simulink model "TosAeb"  (R2021a)
settings: Solver=FixedStepDiscrete  StartTime=0.0  StopTime=inf  FixedStep=0.01  RelTol=1e-3
undefined symbols (not defined in the file or in its folder): ACCModSts, AccSts1, AEB_ObjectMovingOrientation, AEB_ObjectMovingState, CN_BTCActive, CN_CurrentGearR, CN_CurrentGearR_DHT, CN_PTCActive, CN_VDCActive, CN_VSC_EspOff, CN_VSC_FCW_AEB_Error, CN_VSC_FCW_AEB_NoDispay, CN_VSC_FCW_AEB_PerfmDgdtn, CN_VSC_FCW_AEB_TempUnAvlbl, CN_VSC_VehStandstill, CNaccelAvoidMax, CNaccelAvoidMin, CNbrakePedalPress, CNcomfortStrLeftAccelMax, CNcomfortStrLeftAccelMin, CNcomfortStrRightAccelMax, CNcomfortStrRightAccelMin, CNDrvDoorOpen, CNDrvModeEco, CNDrvModeNorm, CNDrvModeOther, CNDrvModeSport, CNDrvSBRreminder, CNNull, CNobjInvalidID, CNPassDoorOpen, CNPassSBRreminder, CNPEBSAccelNegInf, CNPEBSAccelNull, CNPEBSTimeInf, CNPEBSTimeNull, CNSTOPMAX, CNSTOPNULL, CNsubjWidth, CNSwtOFF, CNSwtON, CNTTCMAX, CNTTCNULL, CNVehConfigAEBCycNone, CNVehConfigAEBNone, CNVehConfigAEBPedNone, CNVehConfigFCWNone, CNVehConfigJANone, CollisionSeverity, FALSE, FCWSnvty, JACycObjCalcInfoDefaultParams, JAPedObjCalcInfoDefaultParams, JAVehObjCalcInfoDefaultParams, latCycObjCalcInfoDefaultParams, latPedObjCalcInfoDefaultParams, longObjCalcInfoDefaultParams, ModSts, movingStatus, ObjectType ... (+1112)
libraries: simulink x3031, AEBSFunctionLib x50, Ta_Library x33, AEBSStateMachineLib x23, Mylibrary x12, simulink_extras x7, ObjSelect_lib x3, dspstat3 x2
(part 93 of 102)

== matlab function TosAeb/AEB_Objsel/TA_Processor/AEB_ObjSelect/TargetInfoCheck/Data_precess/TA_EffectCalculate/Obj_FilterFlgCalculate/Lat_ObjFilter/Lat_Objelse/Lat_ObjPre/Subsystem/LatDis_Cal/L2R/L2R_Calculate1 ==
  data: dy input; Valid_Flg output; Width_Large input; Width_half input; dx input; ax input; ay input; vx input; vy input; TTI output; P_TA_PrmForVehLongth input
function [Valid_Flg,TTI] = fcn(dy,Width_Large,Width_half,dx,ax,ay,vx,vy,P_TA_PrmForVehLongth)
%Valid_Flg = false;
%TTI = single(100);
distance_dx = dx;
% 目标处于车辆右侧，无重叠度，向右行驶；
if dy <= single(0) && abs(dy) > Width_Large && vy < single(0)
    Valid_Flg = false;
    TTI = single(100);
% 目标处于车辆右侧，存在重叠，向右行驶
elseif dy <= single(0) && abs(dy) <= Width_Large && vy < single(0)
    distance_y1 = abs(dy) - Width_Large;
    time_1 = solution(single(0.5)*ay,vy,distance_y1);
    distance_x1 = abs(vx)*time_1 + single(0.5)*ax*(time_1)^2;
    time = solution(single(0.5)*ax,vx,distance_dx);
    if distance_x1 <= single(0) || time > time_1
        Valid_Flg = false;
        TTI = single(100);
    else
        if distance_x1 - dx > single(-1) * P_TA_PrmForVehLongth || time <= time_1
            Valid_Flg = true;
            TTI = time;
        else
            Valid_Flg = false;
            TTI = time;
        end
    end
% 目标处于车辆左侧，存在重叠，向右行驶
elseif dy >= single(0) && abs(dy) <= Width_Large && vy < single(0)
    distance_y1 = dy - Width_Large;
    time_1 = solution(single(0.5)*ay,vy,distance_y1);
    distance_x1 = abs(vx)*time_1 + single(0.5)*ax*(time_1)^2;
    time = solution(single(0.5)*ax,vx,distance_dx);
    if distance_x1 <= single(0) || time > time_1
        Valid_Flg = false;
        TTI = single(100);
    else
        if distance_x1 - dx > single(-1)* P_TA_PrmForVehLongth || time <= time_1
            Valid_Flg = true;
            TTI = time;
        else
            Valid_Flg = false;
            TTI = time;
        end
    end
% 目标处于车辆左侧，不存在重叠，向右行驶
elseif dy > single(0) && abs(dy) > Width_Large && vy < single(0)
    distance_y1 = dy - Width_Large;
    distance_y2 = dy + Width_Large;
    time_1 = solution(single(0.5)*ay,vy,distance_y1);
    time_2 = solution(single(0.5)*ay,vy,distance_y2);
    distance_x1 = abs(vx)*time_1 + single(0.5)*ax*(time_1)^2;
    distance_x2 = abs(vx)*time_2 + single(0.5)*ax*(time_2)^2;
    time = solution(single(0.5)*ax,vx,distance_dx);
    if distance_x1 <= single(0) || distance_x2 <= single(0) || time < time_1 -single(1)|| time > time_2 + single(1)
        Valid_Flg = false;
        TTI = single(100);
    else
        if (distance_x1 - dx >= single(0) && distance_x2 - dx <= P_TA_PrmForVehLongth) || (time >= (time_1-single(1)) && time <= (time_2+single(1)))
            Valid_Flg = true;
            TTI = time;
        else
            Valid_Flg = false;
        TTI = single(100);
        end
    end
else
    Valid_Flg = false;
        TTI = single(100);
end
end
%% 一元二次方程求解
function result = solution(a,b,c)
d = single(1) *c;
delta = single(b*b - 4*a*d);
if a == single(0)
    result =  single(c/b);
else
    if delta > single(0)
        r1 = single(((-b) - sqrt(delta))/(2*a));
        r2 = single(((-b) + sqrt(delta))/(2*a));
        if r1 > single(0) && r2 >single(0)
            result = min(r1,r2);
        elseif r1 > single(0) && r2 <= single(0)
            result = r1;
        elseif r1 <= single(0) && r2 > single(0)
            result = r2;
        else
            result = single(100);
        end
    elseif delta == single(0)
        r1 = single((-b)/(2*a));
        r2 = r1;
        if r2 > single(0)
            result = r2;
        else
            result = single(100);
        end
    else
        result = single(100);
    end
end
end
%%

== matlab function TosAeb/AEB_Objsel/TA_Processor/AEB_ObjSelect/TargetInfoCheck/Data_precess/TA_EffectCalculate/Obj_FilterFlgCalculate/Lat_ObjFilter/Lat_Objelse/Lat_ObjPre/Subsystem/LatDis_Cal/R2L/R2L_Calculate ==
  data: dy input; Valid_Flg output; Width_Large input; dx input; ax input; ay input; vx input; vy input; TTI output
function [Valid_Flg,TTI] = fcn(dy,Width_Large,dx,ax,ay,vx,vy)
%Valid_Flg = false;
%TTI = single(100);
distance_dx = dx;
% 目标处于车辆左侧，无重叠度，向左行驶；
if abs(dy) >= Width_Large && dy > single(0) && vy > single(0)
    Valid_Flg = false;
    TTI = single(100);
% 目标处于车辆左侧，存在重叠，向左行驶
elseif dy >= single(0) && abs(dy) < Width_Large && vy > single(0)
    distance_y1 = Width_Large - abs(dy);
    if distance_y1 * vy >single(0)
        distance_y1 = single(-1)*distance_y1;
    else
    end
    time_1 = solution(single(0.5)*ay,vy,distance_y1);
    distance_x1 = abs(vx)*time_1 + single(0.5)*ax*(time_1)^2;
    time = solution(single(0.5)*ax,vx,distance_dx);
    if distance_x1 <= single(0) || time > single(10) || time_1 >single(10)
        Valid_Flg = false;
        TTI = single(100);
    else
       
            Valid_Flg = true;
            TTI = time;
        
    end
% 目标处于车辆右侧，存在重叠，向左行驶
elseif dy <= single(0) && abs(dy) < Width_Large && vy > single(0)
    distance_y1 = Width_Large + dy;
    if distance_y1 * vy >single(0)
        distance_y1 = single(-1)*distance_y1;
    else
    end
    time_1 = solution(single(0.5)*ay,vy,distance_y1);
    distance_x1 = abs(vx)*time_1 + single(0.5)*ax*(time_1)^2;
    time = solution(single(0.5)*ax,vx,distance_dx);
    if distance_x1 <= single(0) || time > single(10) || time_1 >single(10)
        Valid_Flg = false;
        TTI = single(100);
    else
        
            Valid_Flg = true;
            TTI = time;
       
    end
% 目标处于车辆右侧，不存在重叠，向左行驶
elseif dy < single(0) && abs(dy) > Width_Large && vy > single(0)
    distance_y1 = Width_Large + dy;
    distance_y2 = dy - Width_Large;
    time_1 = solution(single(0.5)*ay,vy,distance_y1);
    time_2 = solution(single(0.5)*ay,vy,distance_y2);
    distance_x1 = abs(vx)*time_1 + single(0.5)*ax*(time_1)^2;
    distance_x2 = abs(vx)*time_2 + single(0.5)*ax*(time_2)^2;
    time = solution(single(0.5)*ax,vx,distance_dx);
    if distance_x1 <= single(0) || distance_x2 <= single(0)|| time > single(10) || time_1 >single(10)||time_2 >single(10)
        Valid_Flg = false;
        TTI = single(100);
    else
      
            Valid_Flg = true;
            TTI = time;
        
    end
else
    Valid_Flg = false;
        TTI = single(100);
end
end
%% 一元二次方程求解
function result = solution(a,b,c)
d = single(1) *c;
delta = b*b - 4*a*d;
if abs(a) <= single(0.01) && b ~= single(0)
    result =  abs(c/b);
else
    if delta > single(0)
        r1 = ((-b) - sqrt(delta))/(2*a);
        r2 = ((-b) + sqrt(delta))/(2*a);
        if r1 > single(0) && r2 >single(0)
            result = min(r1,r2);
        elseif r1 > single(0) && r2 <= single(0)
            result = r1;
        elseif r1 <= single(0) && r2 > single(0)
            result = r2;
        else
            result = single(100);
        end
    elseif delta == single(0)
        r1 = (-b)/(2*a);
        r2 = r1;
        if r2 > single(0)
            result = r2;
        else
            result = single(100);
        end
    else
        result = single(100);
    end
end
end
%%

== matlab function TosAeb/AEB_Objsel/TA_Processor/AEB_ObjSelect/TargetInfoCheck/Data_precess/TA_EffectCalculate/Obj_FilterFlgCalculate/Lat_ObjFilter/Lat_Objelse/Lat_ObjPre/Subsystem/LatDis_Cal/R2L/R2L_Calculate1 ==
  data: dy input; Valid_Flg output; Width_Large input; Width_half input; dx input; ax input; ay input; vx input; vy input; TTI output; P_TA_PrmForVehLongth input
function [Valid_Flg,TTI] = fcn(dy,Width_Large,Width_half,dx,ax,ay,vx,vy,P_TA_PrmForVehLongth)
%Valid_Flg = false;
%TTI = single(100);
distance_dx = dx;
% 目标处于车辆左侧，无重叠度，向左行驶；
if abs(dy) >= Width_Large && dy > single(0) && vy > single(0)
    Valid_Flg = false;
    TTI = single(100);
% 目标处于车辆左侧，存在重叠，向左行驶
elseif dy >= single(0) && abs(dy) < Width_Large && vy > single(0)
    distance_y1 = Width_Large - abs(dy);
    time_1 = solution(ay,vy,distance_y1);
    distance_x1 = abs(vx)*time_1 + single(0.5)*ax*(time_1)^2;
    time = solution(ax,vx,distance_dx);
    if distance_x1 <= single(0) || time > time_1
        Valid_Flg = false;
        TTI = single(100);
    else
        if distance_x1 - dx > single(-1) * P_TA_PrmForVehLongth || time <= time_1
            Valid_Flg = true;
            TTI = time;
        else
            Valid_Flg = false;
            TTI = time;
        end
    end
% 目标处于车辆右侧，存在重叠，向左行驶
elseif dy <= single(0) && abs(dy) < Width_Large + single(0.5) && vy > single(0)
    distance_y1 = Width_Large + dy;
    time_1 = solution(ay,vy,distance_y1);
    distance_x1 = abs(vx)*time_1 + single(0.5)*ax*(time_1)^2;
    time = solution(ax,vx,distance_dx);
    if distance_x1 <= single(0) || time > time_1
        Valid_Flg = false;
        TTI = single(100);
    else
        if distance_x1 - dx > single(-1)* P_TA_PrmForVehLongth || time <= time_1
            Valid_Flg = true;
            TTI = time;
        else
            Valid_Flg = false;
            TTI = time;
        end
    end
% 目标处于车辆右侧，不存在重叠，向左行驶
elseif dy < single(0) && abs(dy) > Width_Large && vy > single(0)
    distance_y1 = Width_Large + dy;
    distance_y2 = dy - Width_Large;
    time_1 = solution(ay,vy,distance_y1);
    time_2 = solution(ay,vy,distance_y2);
    distance_x1 = abs(vx)*time_1 + single(0.5)*ax*(time_1)^2;
    distance_x2 = abs(vx)*time_2 + single(0.5)*ax*(time_2)^2;
    time = solution(ax,vx,distance_dx);
    if distance_x1 <= single(0) || distance_x2 <= single(0) || time > time_1 || time < time_2
        Valid_Flg = false;
        TTI = single(100);
    else
       if (distance_x1 - dx >= single(0) && distance_x2 - dx <= P_TA_PrmForVehLongth) || (time <= time_1 && time >= time_2)
            Valid_Flg = true;
            TTI = time;
        else
            Valid_Flg = false;
        TTI = single(100);
        end
    end
else
    Valid_Flg = false;
        TTI = single(100);
end
end
%% 一元二次方程求解
function result = solution(a,b,c)
d = single(1) *c;
delta = b*b - 4*a*d;
if a == single(0)
    result =  c/b;
else
    if delta > single(0)
        r1 = ((-b) - sqrt(delta))/(2*a);
        r2 = ((-b) + sqrt(delta))/(2*a);
        if r1 > single(0) && r2 >single(0)
            result = min(r1,r2);
        elseif r1 > single(0) && r2 <= single(0)
            result = r1;
        elseif r1 <= single(0) && r2 > single(0)
            result = r2;
        else
            result = single(100);
        end
    elseif delta == single(0)
        r1 = (-b)/(2*a);
        r2 = r1;
        if r2 > single(0)
            result = r2;
        else
            result = single(100);
        end
    else
        result = single(100);
    end
end
end
%%

== matlab function TosAeb/AEB_Objsel/TA_Processor/AEB_ObjSelect/TargetInfoCheck/Data_precess/TA_EffectCalculate/Obj_FilterFlgCalculate/Lat_ObjFilter/Lat_Objelse/Lat_ObjPre/Subsystem/LatDis_Cal1/L2R/L2R_Calculate ==
  data: dy input; Valid_Flg output; Width_Large input; Width_half input; dx input; ax input; ay input; vx input; vy input; TTI output; P_TA_PrmForVehLongth input
function [Valid_Flg,TTI] = fcn(dy,Width_Large,Width_half,dx,ax,ay,vx,vy,P_TA_PrmForVehLongth)
%Valid_Flg = false;
%TTI = single(100);
distance_dx = dx;
% 目标处于车辆右侧，无重叠度，向右行驶；
if dy >= Width_Large && vy > single(0)
    Valid_Flg = false;
    TTI = single(100);
% 目标处于车辆右侧，存在重叠，向右行驶
elseif dy >= single(0) && dy < Width_Large && vy > single(0)
    distance_y1 = abs(dy) - Width_Large;
    time_1 = solution(ay,vy,distance_y1);
    distance_x1 = abs(vx)*time_1 + single(0.5)*ax*(time_1)^2;
    time = solution(ax,vx,distance_dx);
    if distance_x1 <= single(0) || time > time_1
        Valid_Flg = false;
        TTI = single(100);
    else
        if distance_x1 - dx > single(-1) * P_TA_PrmForVehLongth || time <= time_1
            Valid_Flg = true;
            TTI = time;
        else
            Valid_Flg = false;
            TTI = time;
        end
    end
% 目标处于车辆左侧，存在重叠，向右行驶
elseif dy <= single(0) && abs(dy) < Width_Large && vy > single(0)
    distance_y1 = dy - Width_Large;
    time_1 = solution(ay,vy,distance_y1);
    distance_x1 = abs(vx)*time_1 + single(0.5)*ax*(time_1)^2;
    time = solution(ax,vx,distance_dx);
    if distance_x1 <= single(0) || time > time_1
        Valid_Flg = false;
        TTI = single(100);
    else
        if distance_x1 - dx > single(-1)* P_TA_PrmForVehLongth || time <= time_1
            Valid_Flg = true;
            TTI = time;
        else
            Valid_Flg = false;
            TTI = time;
        end
    end
% 目标处于车辆左侧，不存在重叠，向右行驶
elseif dy < single(0) && abs(dy) > Width_Large && vy > single(0)
    distance_y1 = dy - Width_Large;
    distance_y2 = dy + Width_Large;
    time_1 = solution(ay,vy,distance_y1);
    time_2 = solution(ay,vy,distance_y2);
    distance_x1 = abs(vx)*time_1 + single(0.5)*ax*(time_1)^2;
    distance_x2 = abs(vx)*time_2 + single(0.5)*ax*(time_2)^2;
    time = solution(ax,vx,distance_dx);
    if distance_x1 <= single(0) || distance_x2 <= single(0) || time > time_1 || time < time_2
        Valid_Flg = false;
        TTI = single(100);
    else
        if (distance_x1 - dx >= single(0) && distance_x2 - dx <= P_TA_PrmForVehLongth) || (time <= time_1 && time >= time_2)
            Valid_Flg = true;
            TTI = time;
        else
            Valid_Flg = false;
        TTI = single(100);
        end
    end
else
    Valid_Flg = false;
        TTI = single(100);
end
end
%% 一元二次方程求解
function result = solution(a,b,c)
d = single(1) *c;
delta = single(b*b - 4*a*d);
if a == single(0)
    result =  single(c/b);
else
    if delta > single(0)
        r1 = single(((-b) - sqrt(delta))/(2*a));
        r2 = single(((-b) + sqrt(delta))/(2*a));
        if r1 > single(0) && r2 >single(0)
            result = min(r1,r2);
        elseif r1 > single(0) && r2 <= single(0)
            result = r1;
        elseif r1 <= single(0) && r2 > single(0)
            result = r2;
        else
            result = single(100);
        end
    elseif delta == single(0)
        r1 = single((-b)/(2*a));
        r2 = r1;
        if r2 > single(0)
            result = r2;
        else
            result = single(100);
        end
    else
        result = single(100);
    end
end
end
%%

== stateflow chart TosAeb/FcwAebJa_Step_sys/ALG_AEBSystem/ALG_AEBController/AEB_FuncImpManagementApp/FuncManeuArbCalc/brkFuncManeuArbCalc/axTgtReqValueCalc1/axTgtReqValueCalc4AebControl/JerkFuncIncCalc/IncPhaseCalc/Chart ==
  state IncrementStage [function]
    (default) -> (junction 173)
    (junction 173) -> (junction 167) [order 1] : [aErrorDesiredTgtValue < 0]
    (junction 167) -> (junction 175)
    (junction 173) -> (junction 179) [order 2] : {
        |    time4IncControlling = 0;
        |   currentIncStage = 0;
        | }
    (junction 175) -> (junction 171) [order 1] : [aErrorDesiredTgtValue <...
        | P_AEB_FIM_aErrorThres4BrakeStage5]
    (junction 175) -> (junction 170) [order 2]
    (junction 171) -> (junction 166) : {
        |   setStage(5);
        |   currentIncStage = 5;
        | }
    (junction 170) -> (junction 174) [order 1] : [aErrorDesiredTgtValue <...
        |  P_AEB_FIM_aErrorThres4BrakeStage4]
    (junction 170) -> (junction 168) [order 2]
    (junction 174) -> (junction 172) : {
        |    setStage(4);
        |    currentIncStage = 4;
        | }
    (junction 168) -> (junction 150) [order 1] : [aErrorDesiredTgtValue <...
        |  P_AEB_FIM_aErrorThres4BrakeStage3]
    (junction 168) -> (junction 169) [order 2]
    (junction 150) -> (junction 151) : {
        |    setStage(3);
        |    currentIncStage = 3;
        | }
    (junction 169) -> (junction 138) [order 1] : [aErrorDesiredTgtValue <...
        |  P_AEB_FIM_aErrorThres4BrakeStage2]
    (junction 169) -> (junction 146) [order 2]
    (junction 138) -> (junction 137) : {
        |    setStage(2);
        |    currentIncStage = 2;
        | }
    (junction 146) -> (junction 141) [order 1] : [aErrorDesiredTgtValue <...
        |  P_AEB_FIM_aErrorThres4BrakeStage1]
    (junction 146) -> (junction 145) [order 2]
    (junction 141) -> (junction 143) : {
        |    setStage(1) ;
        |    currentIncStage = 1;
        | }
    (junction 145) -> (junction 179)
    (junction 143) -> (junction 145)
    (junction 137) -> (junction 143)
    (junction 151) -> (junction 137)
    (junction 172) -> (junction 151)
    (junction 166) -> (junction 172)
    (junction 179) -> (junction 178)
  state setStage(Stage) [function]
    (default) -> (junction 183)
    (junction 183) -> (junction 185) [order 1] : [Stage > ReachedIncStage]
    (junction 185) -> (junction 187) : {
        |   ReachedIncStage = Stage;
        | }
    (junction 183) -> (junction 189) [order 2]
    (junction 187) -> (junction 189)
    (junction 189) -> (junction 192)
  state IncrementTimer(CountStage) [function]
    (default) -> (junction 92)
    (junction 92) -> (junction 98) : {
        |   index=0;
        | }
    (junction 98) -> (junction 94) [order 1] : [index<CountStage]
    (junction 97) -> (junction 98)
    (junction 98) -> (junction 96) [order 2]
    (junction 94) -> (junction 95) : {
        |    time4IncControlling += Ts;
        | }
    (junction 95) -> (junction 97) : {index=index+1;}
  (default) -> (junction 199)
  (junction 199) -> (junction 202) [order 1] : [AebActiveFirstCycle == true]
  (junction 199) -> (junction 201) [order 2]
  (junction 202) -> (junction 205) : {
      |    ReachedIncStage = 0;
      |    currentIncStage = 0;
      |    time4IncControlling = 0;
      | }
  (junction 205) -> (junction 201)
  (junction 201) -> (junction 53) : {
      |    IncrementStage();
      | }
  (junction 53) -> (junction 58) [order 1] : [ReachedIncStage > ...
      | currentIncStage]
  (junction 53) -> (junction 62) [order 2] : {
      |   IncrementTimer(currentIncStage);
      | }
  (junction 58) -> (junction 60)
  (junction 60) -> (junction 62)
  (junction 62) -> (junction 65)
  (junction 65) -> (junction 101) [order 1] : [dxFrontBumperPredicted < P_AEB_FIM_distMinThres4LowControlling]
  (junction 65) -> (junction 105) [order 2]
  (junction 101) -> (junction 103)
  (junction 103) -> (junction 110) [order 1] : [aErrorDesiredTgtValue < P_AEB_FIM_aDeltaThres4BrakeStage6]
  (junction 103) -> (junction 115) [order 2]
  (junction 110) -> (junction 113) : {
      |   IncrementTimer(uint8(2*P_AEB_FIM_TimerNumber4JerkInc));
      | }
  (junction 115) -> (junction 117) [order 1] : [aErrorDesiredTgtValue < P_AEB_FIM_aErrorThres4BrakeStage5]
  (junction 115) -> (junction 122) [order 2]
  (junction 117) -> (junction 119) : {
      |    IncrementTimer(P_AEB_FIM_TimerNumber4JerkInc);
      | }
  (junction 122) -> (junction 105)
  (junction 119) -> (junction 122)
  (junction 113) -> (junction 119)
  (junction 105) -> (junction 108)
  data: Stage function_input uint8; CountStage function_input uint8; aErrorDesiredTgtValue input; dxFrontBumperPredicted input; AebActiveFirstCycle input; Ts input; P_AEB_FIM_aErrorThres4BrakeStage1 input; P_AEB_FIM_aErrorThres4BrakeStage2 input; P_AEB_FIM_aErrorThres4BrakeStage3 input; P_AEB_FIM_aErrorThres4BrakeStage4 input; P_AEB_FIM_aErrorThres4BrakeStage5 input; ReachedIncStage output uint8 =0; currentIncStage output uint8 =0; time4IncControlling output single =0; index local uint8; P_AEB_FIM_aDeltaThres4BrakeStage6 input; P_AEB_FIM_distMinThres4LowControlling input; P_AEB_FIM_TimerNumber4JerkInc input

== stateflow chart TosAeb/FcwAebJa_Step_sys/ALG_AEBSystem/ALG_AEBController/AEB_FuncImpManagementApp/FuncManeuArbCalc/brkFuncManeuArbCalc/axTgtReqValueCalc1/axTgtReqValueCalc4AebControl/aDesiredCalc/If Action Subsystem1/accelAvoidLowLvlBrkCalc/AccelAvoidSituationJudge ==
  (default) -> (junction 16)
  (junction 16) -> (junction 17) [order 1] : [vx < 0]
  (junction 16) -> (junction 27) [order 2]
  (junction 17) -> (junction 74)
  (junction 74) -> (junction 76) [order 1] : [axAbsObj  < 0]
  (junction 27) -> (junction 29) [order 1] : [ axAbsObj  < 0]
  (junction 74) -> (junction 25) [order 2] : {
      | 	send(Situation2);
      | }
  (junction 76) -> (junction 19) : {
      | 	send(Situation1);
      | }
  (junction 27) -> (junction 33) [order 2] : {
      | 	send(Situation4);
      | }
  (junction 29) -> (junction 31) : {
      | 	send(Situation3);
      | }
  (junction 31) -> (junction 33)
  (junction 25) -> (junction 31)
  (junction 19) -> (junction 25)
  (junction 33) -> (junction 86)
  data: vx input; axAbsObj input
  events: Situation1 output FUNCTION_CALL_EVENT; Situation2 output FUNCTION_CALL_EVENT; Situation3 output FUNCTION_CALL_EVENT; Situation4 output FUNCTION_CALL_EVENT

== stateflow chart TosAeb/FcwAebJa_Step_sys/ALG_AEBSystem/ALG_AEBController/AEB_FuncImpManagementApp/FuncManeuArbCalc/brkFuncManeuArbCalc/axTgtReqValueCalc1/axTgtReqValueCalc4AebControl/aDesiredCalc/If Action Subsystem1/tObjStopCalc/StopTimeFlow ==
  state temptStop = tStopFuncCalc(velRel,accelRel) [function]
  (default) -> (junction 2)
  (junction 2) -> (junction 3) [order 1] : [accelRel == 0]
  (junction 2) -> (junction 14) [order 2]
  (junction 3) -> (junction 5)
  (junction 14) -> (junction 16) [order 1] : [(accelRel > 0 && velRel <= 0)||...
      | (accelRel < 0 && velRel >= 0)]
  (junction 5) -> (junction 7) [order 1] : [velRel == 0]
  (junction 14) -> (junction 20) [order 2] : {
      | 	tStop = CNSTOPMAX;
      |  }
  (junction 16) -> (junction 18) : {
      |
      | 	tStop = tStopFuncCalc(velRel,accelRel);
      | }
  (junction 5) -> (junction 11) [order 2] : {
      | 	tStop = CNSTOPMAX;
      | }
  (junction 7) -> (junction 9) : {
      | 	tStop = CNNull;
      | }
  (junction 18) -> (junction 20)
  (junction 11) -> (junction 18)
  (junction 9) -> (junction 11)
  (junction 20) -> (junction 24)
  data: velRel function_input; accelRel function_input; temptStop function_output; tStop output; CNNull parameter double; CNSTOPMAX parameter double; accelRel input; velRel input

== stateflow chart TosAeb/FcwAebJa_Step_sys/ALG_AEBSystem/ALG_AEBController/AEB_FuncImpManagementApp/FuncManeuArbCalc/brkFuncManeuArbCalc/axTgtReqValueCalc1/axTgtReqValueCalc4AebControl/axTgtReqValueCalc/axTgtReqValueFinal/axTgtReqValueCalc/axTgtReqValueCalc/axTgtReqValueFlow ==
  (default) -> (junction 7)
  (junction 7) -> (junction 9) [order 1] : [abs(aErrorADesiredAxTgtReq) <= 1e-6]
  (junction 9) -> (junction 11) : {
      |    axTgtReqValue = ...
      |        accelAvoid;
      | }
  (junction 7) -> (junction 21) [order 2]
  (junction 21) -> (junction 23) [order 1] : [aErrorADesiredAxTgtReq < 0]
  (junction 21) -> (junction 25) [order 2]
  (junction 23) -> (junction 13)
  (junction 13) -> (junction 15) [order 1] : [aErrorADesiredAxTgtReq < JerkDecTimeSlot]
  (junction 13) -> (junction 19) [order 2] : {
      |   axTgtReqValue = ...
      |         axTgtReqValueK1 + aErrorADesiredAxTgtReq;
      | }
  (junction 15) -> (junction 17) : {
      |    axTgtReqValue = ...
      |          axTgtReqValueK1 + JerkDecTimeSlot;
      | }
  (junction 25) -> (junction 27) [order 1] : [aErrorADesiredAxTgtReq > JerkIncTimeSlot]
  (junction 25) -> (junction 31) [order 2] : {
      |   axTgtReqValue =...
      |         axTgtReqValueK1 + aErrorADesiredAxTgtReq;
      | }
  (junction 27) -> (junction 29) : {
      |   axTgtReqValue = ...
      |         axTgtReqValueK1 + JerkIncTimeSlot;
      | }
  (junction 11) -> (junction 17)
  (junction 29) -> (junction 31)
  (junction 19) -> (junction 29)
  (junction 17) -> (junction 19)
  (junction 31) -> (junction 38)
  (junction 38) -> (junction 40) [order 1] : [accelMax < accelMin]
  (junction 38) -> (junction 44) [order 2] : {
      | axTgtReqValue = min(max(axTgtReqValue, accelMin), accelMax);
      | }
  (junction 40) -> (junction 42) : {
      |   axTgtReqValue = accelMin;
      | }
  (junction 42) -> (junction 44)
  (junction 44) -> (junction 49)
  data: JerkIncTimeSlot input; JerkDecTimeSlot input; aErrorADesiredAxTgtReq input; accelAvoid input; accelMax input; accelMin input; axTgtReqValueK1 input; axTgtReqValue output

== stateflow chart TosAeb/FcwAebJa_Step_sys/ALG_AEBSystem/ALG_AEBController/AEB_FuncImpManagementApp/FuncManeuArbCalc/brkFuncManeuArbCalc/axTgtReqValueCalc1/axTgtReqValueCalc4AebControl/axTgtReqValueCalc/axTgtReqValueFinal1/axTgtReqValueCalc/axTgtReqValueCalc/axTgtReqValueFlow ==
  (default) -> (junction 7)
  (junction 7) -> (junction 9) [order 1] : [abs(aErrorADesiredAxTgtReq) <= 1e-6]
  (junction 9) -> (junction 11) : {
      |    axTgtReqValue = ...
      |        accelAvoid;
      | }
  (junction 7) -> (junction 21) [order 2]
  (junction 21) -> (junction 23) [order 1] : [aErrorADesiredAxTgtReq < 0]
  (junction 21) -> (junction 25) [order 2]
  (junction 23) -> (junction 13)
  (junction 13) -> (junction 15) [order 1] : [aErrorADesiredAxTgtReq < JerkDecTimeSlot]
  (junction 13) -> (junction 19) [order 2] : {
      |   axTgtReqValue = ...
      |         axTgtReqValueK1 + aErrorADesiredAxTgtReq;
      | }
  (junction 15) -> (junction 17) : {
      |    axTgtReqValue = ...
      |          axTgtReqValueK1 + JerkDecTimeSlot;
      | }
  (junction 25) -> (junction 27) [order 1] : [aErrorADesiredAxTgtReq > JerkIncTimeSlot]
  (junction 25) -> (junction 31) [order 2] : {
      |   axTgtReqValue =...
      |         axTgtReqValueK1 + aErrorADesiredAxTgtReq;
      | }
  (junction 27) -> (junction 29) : {
      |   axTgtReqValue = ...
      |         axTgtReqValueK1 + JerkIncTimeSlot;
      | }
  (junction 11) -> (junction 17)
  (junction 29) -> (junction 31)
  (junction 19) -> (junction 29)
  (junction 17) -> (junction 19)
  (junction 31) -> (junction 38)
  (junction 38) -> (junction 40) [order 1] : [accelMax < accelMin]
  (junction 38) -> (junction 44) [order 2] : {
      | axTgtReqValue = min(max(axTgtReqValue, accelMin), accelMax);
      | }
  (junction 40) -> (junction 42) : {
      |   axTgtReqValue = accelMin;
      | }
  (junction 42) -> (junction 44)
  (junction 44) -> (junction 49)
  data: JerkIncTimeSlot input; JerkDecTimeSlot input; aErrorADesiredAxTgtReq input; accelAvoid input; accelMax input; accelMin input; axTgtReqValueK1 input; axTgtReqValue output

== stateflow chart TosAeb/FcwAebJa_Step_sys/ALG_AEBSystem/ALG_AEBController/AEB_FuncImpManagementApp/FuncManeuArbCalc/brkFuncManeuArbCalc/axTgtReqValueCalc1/axTgtReqValueCalc4AebFull/BrkFuncDataManagement ==
  state axTgtReqValue = AEB_TgtDecelFunc(maxDecel,maxDecelGredient,axTgtReqValueK1) [function]
  state MaxDecel = VehAEBfullMaxDecelLookupTable(subjVel) [function]
  state MaxDecel = VehAEBpartMaxDecelLookupTable(subjVel) [function]
  state LongFuncAEBTrigger [function]
    (default) -> (junction 231)
    (junction 231) -> (junction 234) [order 1] : [AEBpartFuncImpArb == ModSts.ActiveState]
    (junction 231) -> (junction 232) [order 2]
    (junction 234) -> (junction 242) : {
        | 	maxDecel = P_FIM_VehAEBPartDecelMinThres;
        | 	maxDecelGredient = P_FIM_VehAEBPartDecelGradientThres;
        | 	axTgtReqValue = AEB_TgtDecelFunc(maxDecel,maxDecelGredient,axTgtReqValueK1);
        | }
    (junction 242) -> (junction 236)
    (junction 236) -> (junction 237)
    (junction 232) -> (junction 241) [order 1] : [(AEBfullFuncImpArb == ModSts.ActiveState&&...
        | P_AEB_FIM_BrkControllerConfig == false)]
    (junction 232) -> (junction 254) [order 2]
    (junction 241) -> (junction 239)
    (junction 239) -> (junction 240) [order 1] : [longTgt_MovingState == AEB_ObjectMovingState.Still]
    (junction 240) -> (junction 233) : {
        | 	maxDecel = P_FIM_VehAEBfullDecelMinThresSO;
        | 	maxDecelGredient = ...
        |       P_FIM_VehAEBfullDecelGradientThresSO;
        | }
    (junction 239) -> (junction 235) [order 2] : {
        |
        | 	maxDecel =  ...
        | 	VehAEBfullMaxDecelLookupTable(subjVel);
        | 	maxDecelGredient = ...
        |       P_FIM_VehAEBfullDecelGradientThresMO;
        | }
    (junction 233) -> (junction 235)
    (junction 235) -> (junction 243) : {
        | 	axTgtReqValue = AEB_TgtDecelFunc(maxDecel,maxDecelGredient,axTgtReqValueK1);
        | }
    (junction 243) -> (junction 237)
    (junction 237) -> (junction 250)
    (junction 254) -> (junction 244) [order 1] : [EBAFuncImpArb == ModSts.ActiveState]
    (junction 254) -> (junction 273) [order 2]
    (junction 244) -> (junction 257) : {
        | 	maxDecel = P_FIM_VehEBADecelMinThres;
        | 	maxDecelGredient = P_FIM_VehEBADecelGradientThres;
        | 	axTgtReqValue = AEB_TgtDecelFunc(maxDecel,maxDecelGredient,axTgtReqValueK1);
        | }
    (junction 257) -> (junction 250)
    (junction 250) -> (junction 274)
    (junction 274) -> (junction 273)
    (junction 273) -> (junction 276)
  state LatFuncTrigger [function]
    (default) -> (junction 287)
    (junction 287) -> (junction 281) [order 1] : [cycAEBpartFuncImpArb == ModSts.ActiveState]
    (junction 287) -> (junction 284) [order 2]
    (junction 281) -> (junction 282) : {
        | 	maxDecel = VehAEBpartMaxDecelLookupTable(subjVel);
        | 	maxDecelGredient = P_FIM_CycAEBPartDecelGradientThres;
        | 	axTgtReqValue = AEB_TgtDecelFunc(maxDecel,maxDecelGredient,axTgtReqValueK1);
        | }
    (junction 282) -> (junction 290)
    (junction 290) -> (junction 292)
    (junction 284) -> (junction 285) [order 1] : [cycAEBfullFuncImpArb == ModSts.ActiveState]
    (junction 284) -> (junction 288) [order 2]
    (junction 285) -> (junction 286) : {
        | 	maxDecel = P_FIM_CycAEBfullDecelMinThres;
        | 	maxDecelGredient = P_FIM_CycAEBfullDecelGradientThres;
        | 	axTgtReqValue = AEB_TgtDecelFunc(maxDecel,maxDecelGredient,axTgtReqValueK1);
        | }
    (junction 286) -> (junction 292)
    (junction 292) -> (junction 298)
    (junction 288) -> (junction 289) [order 1] : [pedAEBfullFuncImpArb == ModSts.ActiveState]
    (junction 288) -> (junction 320) [order 2]
    (junction 289) -> (junction 291) : {
        | 	maxDecel = P_FIM_PedAEBfullDecelMinThres;
        | 	maxDecelGredient = P_FIM_PedAEBfullDecelGradientThres;
        | 	axTgtReqValue = AEB_TgtDecelFunc(maxDecel,maxDecelGredient,axTgtReqValueK1);
        | }
    (junction 291) -> (junction 441)
    (junction 441) -> (junction 320)
    (junction 298) -> (junction 441)
    (junction 320) -> (junction 324)
  state FCTBFuncTrigger [function]
    (default) -> (junction 425)
    (junction 425) -> (junction 431) [order 1] : [FCTBFuncImpArb == ModSts.ActiveState]
    (junction 425) -> (junction 432) [order 2]
    (junction 431) -> (junction 443) : {
        | 	%maxDecel = P_FIM_FCTBDecelMinThres;
        | 	%maxDecelGredient = P_FIM_FCTBDecelGradientThres;
        | 	axTgtReqValue = FCTB_BrkgReqVal;
        | }
    (junction 443) -> (junction 424)
    (junction 424) -> (junction 432)
    (junction 432) -> (junction 438)
  state JaFuncTrigger [function]
    (default) -> (junction 365)
    (junction 365) -> (junction 362) [order 1] : [JA_PTW_AEBFuncImpArb == ModSts.ActiveState]
    (junction 365) -> (junction 367) [order 2]
    (junction 362) -> (junction 361)
    (junction 361) -> (junction 385) : {
        | 	maxDecel = P_FIM_JA_PTW_AEBDecelMinThres;
        | 	maxDecelGredient = P_FIM_JA_PTW_AEBDecelGradientThres;
        | 	axTgtReqValue = AEB_TgtDecelFunc(maxDecel,maxDecelGredient,axTgtReqValueK1);
        | }
    (junction 385) -> (junction 366)
    (junction 367) -> (junction 363) [order 1] : [JA_cycAEBFuncImpArb == ModSts.ActiveState]
    (junction 367) -> (junction 359) [order 2]
    (junction 363) -> (junction 358)
    (junction 358) -> (junction 366) : {
        | 	maxDecel = P_FIM_JA_cycAEBDecelMinThres;
        | 	maxDecelGredient = P_FIM_JA_cycAEBDecelGradientThres;
        | 	axTgtReqValue = AEB_TgtDecelFunc(maxDecel,maxDecelGredient,axTgtReqValueK1);
        | }
    (junction 366) -> (junction 360)
    (junction 359) -> (junction 368) [order 1] : [JA_pedAEBFuncImpArb == ModSts.ActiveState]
    (junction 359) -> (junction 453) [order 2]
    (junction 368) -> (junction 364)
    (junction 364) -> (junction 360) : {
        | 	maxDecel = P_FIM_JA_pedAEBDecelMinThres;
        | 	maxDecelGredient = P_FIM_JA_pedAEBDecelGradientThres;
        | 	axTgtReqValue = AEB_TgtDecelFunc(maxDecel,maxDecelGredient,axTgtReqValueK1);
        | }
    (junction 360) -> (junction 447)
    (junction 453) -> (junction 448) [order 1] : [JA_AEBfullFuncImpArb == ModSts.ActiveState]
    (junction 453) -> (junction 386) [order 2]
    (junction 448) -> (junction 449) : {
        | 	maxDecel = P_FIM_JAAEBfullDecelMinThres;
        | 	maxDecelGredient = P_FIM_JAAEBfullDecelGradientThres;
        | 	axTgtReqValue = AEB_TgtDecelFunc(maxDecel,maxDecelGredient,axTgtReqValueK1);
        | }
    (junction 449) -> (junction 447)
    (junction 447) -> (junction 387)
    (junction 387) -> (junction 386)
    (junction 386) -> (junction 390)
  (default) -> (junction 44)
  (junction 44) -> (junction 45) [order 1] : [AEBpartFuncImpArb == ModSts.ActiveState||...
      | (AEBfullFuncImpArb == ModSts.ActiveState&&...
      | P_AEB_FIM_BrkControllerConfig == false)||...
      | EBAFuncImpArb == ModSts.ActiveState]
  (junction 44) -> (junction 48) [order 2]
  (junction 45) -> (junction 46) : {
      | 	LongFuncAEBTrigger();
      | }
  (junction 46) -> (junction 47)
  (junction 47) -> (junction 51)
  (junction 48) -> (junction 49) [order 1] : [cycAEBpartFuncImpArb == ModSts.ActiveState||...
      | cycAEBfullFuncImpArb == ModSts.ActiveState||...
      | pedAEBfullFuncImpArb == ModSts.ActiveState]
  (junction 48) -> (junction 56) [order 2]
  (junction 49) -> (junction 50) : {
      | 	LatFuncTrigger();
      | }
  (junction 50) -> (junction 51)
  (junction 51) -> (junction 59)
  (junction 56) -> (junction 57) [order 1] : [FCTBFuncImpArb == ModSts.ActiveState &&...
      | IsNeedBrake == true]
  (junction 56) -> (junction 192) [order 2]
  (junction 57) -> (junction 58) : {
      | 	FCTBFuncTrigger();
      | }
  (junction 192) -> (junction 194) [order 1] : [JA_PTW_AEBFuncImpArb == ModSts.ActiveState||...
      | JA_cycAEBFuncImpArb == ModSts.ActiveState||...
      | JA_pedAEBFuncImpArb == ModSts.ActiveState||...
      | JA_AEBfullFuncImpArb == ModSts.ActiveState]
  (junction 192) -> (junction 60) [order 2] : {
      | 	maxDecel = 0;
      | 	maxDecelGredient = 0;
      | 	axTgtReqValue = 0;
      |
      | }
  (junction 194) -> (junction 196)
  (junction 58) -> (junction 59)
  (junction 59) -> (junction 198)
  (junction 196) -> (junction 198) : {
      | 	JaFuncTrigger();
      | }
  (junction 198) -> (junction 88)
  (junction 88) -> (junction 60)
  (junction 60) -> (junction 62)
  data: maxDecel function_input single; maxDecelGredient function_input single; axTgtReqValue function_output; axTgtReqValueK1 function_input single; subjVel function_input single; MaxDecel function_output; subjVel function_input single; MaxDecel function_output; maxDecelGredient output single; AEBpartFuncImpArb input Enum: ModSts; cycAEBpartFuncImpArb input Enum: ModSts; AEBfullFuncImpArb input Enum: ModSts; JA_AEBfullFuncImpArb input Enum: ModSts; cycAEBfullFuncImpArb input Enum: ModSts; pedAEBfullFuncImpArb input Enum: ModSts; EBAFuncImpArb input Enum: ModSts; FCTBFuncImpArb input Enum: ModSts; JA_PTW_AEBFuncImpArb input Enum: ModSts; JA_cycAEBFuncImpArb input Enum: ModSts; JA_pedAEBFuncImpArb input Enum: ModSts; subjVel input single; longTgt_MovingState input Enum: AEB_ObjectMovingState; IsNeedBrake input; FCTB_BrkgReqVal input; axTgtReqValueK1 input single; maxDecel output single; axTgtReqValue output single; P_FIM_VehAEBPartDecelGradientThres input; P_FIM_VehAEBPartDecelMinThres input; P_FIM_CycAEBPartDecelGradientThres input; P_FIM_VehAEBfullDecelGradientThresMO input; P_FIM_VehAEBfullDecelGradientThresSO input; P_FIM_VehAEBfullDecelMinThresSO input; P_FIM_CycAEBfullDecelGradientThres input; P_FIM_CycAEBfullDecelMinThres input; P_FIM_PedAEBfullDecelGradientThres input; P_FIM_PedAEBfullDecelMinThres input; P_FIM_FCTBDecelGradientThres input; P_FIM_FCTBDecelMinThres input; P_FIM_JAAEBfullDecelGradientThres input; P_FIM_JAAEBfullDecelMinThres input; P_FIM_VehEBADecelGradientThres input; P_FIM_VehEBADecelMinThres input; P_FIM_JA_PTW_AEBDecelGradientThres input; P_FIM_JA_PTW_AEBDecelMinThres input; P_FIM_JA_cycAEBDecelGradientThres input; P_FIM_JA_cycAEBDecelMinThres input; P_FIM_JA_pedAEBDecelGradientThres input; P_FIM_JA_pedAEBDecelMinThres input; P_AEB_FIM_BrkControllerConfig input

== stateflow chart TosAeb/FcwAebJa_Step_sys/ALG_AEBSystem/ALG_AEBController/AEB_FuncImpManagementApp/FuncManeuArbCalc/brkFuncManeuArbCalc/axTgtReqValueManeuCalc/AEBTriggerManeuCalc1 ==
  state AEBQuitState
    en:
    timeCounter = 0;
    AEBTriggerManeu = true;
    %axTgtReqValueIntial = axTgtReqValue;
    du:
    AEBTriggerManeu = true;
    timeCounter += Ts;
    axTgtReqValueManeu = axTgtReqValueOptCalc(Ts,axJerkQuit,axTgtReqValueIntial);
  state AEBNormalState
    en,du:
    timeCounter = 0;
    AEBTriggerManeu= AEBTrigger;
    axTgtReqValueManeu = axTgtReqValue;
    exit:
    axTgtReqValueIntial = axTgtReqValueK1;
  state axTgtReqValueManeu = axTgtReqValueOptCalc(Ts,axJerkQuit,axTgtReqValueIntial) [function]
  (default) -> (junction 7)
  (junction 7) -> AEBNormalState [order 2]
  (junction 7) -> AEBQuitState [order 1] : [AEBQuitFlg == true]
  AEBQuitState -> AEBNormalState : [timeCounter >= PmaxQuitTime || ...
      | abs(axTgtReqValueManeu) < 1e-6]
  AEBNormalState -> AEBQuitState : [AEBQuitFlg == true]
  data: Ts function_input single; axJerkQuit function_input single; axTgtReqValueIntial function_input single; axTgtReqValueManeu function_output; AEBQuitFlg input; AEBTrigger input; axTgtReqValue input single; axTgtReqValueK1 input single; Ts input single; timeCounter local single; PmaxQuitTime input single; AEBTriggerManeu output boolean; axTgtReqValueManeu output single; axTgtReqValueIntial local single; axJerkQuit input single

== matlab function TosAeb/AEB_Objsel/TA_Processor/AEB_ObjSelect/TargetInfoCheck/Data_precess/TA_EffectCalculate/Obj_FilterFlgCalculate/Lat_ObjFilter/Lat_Objelse/Lat_ObjPre/Subsystem/LatDis_Cal1/R2L/R2L_Calculate ==
  data: dy input; Valid_Flg output; Width_Large input; Width_half input; dx input; ax input; ay input; vx input; vy input; TTI output; P_TA_PrmForVehLongth input
function [Valid_Flg,TTI] = fcn(dy,Width_Large,Width_half,dx,ax,ay,vx,vy,P_TA_PrmForVehLongth)
%Valid_Flg = false;
%TTI = single(100);
distance_dx = dx;
% 目标处于车辆左侧，无重叠度，向左行驶；
if abs(dy) >= Width_Large && dy < single(0) && vy > single(0)
    Valid_Flg = false;
    TTI = single(100);
% 目标处于车辆左侧，存在重叠，向左行驶
elseif dy <= single(0) && abs(dy) < Width_Large && vy > single(0)
    distance_y1 = Width_Large - abs(dy);
    time_1 = solution(ay,vy,distance_y1);
    distance_x1 = abs(vx)*time_1 + single(0.5)*ax*(time_1)^2;
    time = solution(ax,vx,distance_dx);
    if distance_x1 <= single(0) || time > time_1
        Valid_Flg = false;
        TTI = single(100);
    else
        if distance_x1 - dx > single(-1) * P_TA_PrmForVehLongth || time <= time_1
            Valid_Flg = true;
            TTI = time;
        else
            Valid_Flg = false;
            TTI = time;
        end
    end
% 目标处于车辆右侧，存在重叠，向左行驶
elseif dy >= single(0) && dy < Width_Large && vy > single(0)
    distance_y1 = Width_Large + dy;
    time_1 = solution(ay,vy,distance_y1);
    distance_x1 = abs(vx)*time_1 + single(0.5)*ax*(time_1)^2;
    time = solution(ax,vx,distance_dx);
    if distance_x1 <= single(0) || time > time_1
        Valid_Flg = false;
        TTI = single(100);
    else
        if distance_x1 - dx > single(-1)* P_TA_PrmForVehLongth || time <= time_1
            Valid_Flg = true;
            TTI = time;
        else
            Valid_Flg = false;
            TTI = time;
        end
    end
% 目标处于车辆右侧，不存在重叠，向左行驶
elseif dy > single(0) && abs(dy) > Width_Large && vy > single(0)
    distance_y1 = Width_Large + dy;
    distance_y2 = dy - Width_Large;
    time_1 = solution(ay,vy,distance_y1);
    time_2 = solution(ay,vy,distance_y2);
    distance_x1 = abs(vx)*time_1 + single(0.5)*ax*(time_1)^2;
    distance_x2 = abs(vx)*time_2 + single(0.5)*ax*(time_2)^2;
    time = solution(ax,vx,distance_dx);
    if distance_x1 <= single(0) || distance_x2 <= single(0) || time > time_1 || time < time_2
        Valid_Flg = false;
        TTI = single(100);
    else
       if (distance_x1 - dx >= single(0) && distance_x2 - dx <= P_TA_PrmForVehLongth) || (time <= time_1 && time >= time_2)
            Valid_Flg = true;
            TTI = time;
        else
            Valid_Flg = false;
        TTI = single(100);
        end
    end
else
    Valid_Flg = false;
        TTI = single(100);
end
end
%% 一元二次方程求解
function result = solution(a,b,c)
d = single(1) *c;
delta = b*b - 4*a*d;
if a == single(0)
    result =  c/b;
else
    if delta > single(0)
        r1 = ((-b) - sqrt(delta))/(2*a);
        r2 = ((-b) + sqrt(delta))/(2*a);
        if r1 > single(0) && r2 >single(0)
            result = min(r1,r2);
        elseif r1 > single(0) && r2 <= single(0)
            result = r1;
        elseif r1 <= single(0) && r2 > single(0)
            result = r2;
        else
            result = single(100);
        end
    elseif delta == single(0)
        r1 = (-b)/(2*a);
        r2 = r1;
        if r2 > single(0)
            result = r2;
        else
            result = single(100);
        end
    else
        result = single(100);
    end
end
end
%%

== matlab function TosAeb/AEB_Objsel/TA_Processor/AEB_ObjSelect/TargetInfoCheck/Data_precess/TA_EffectCalculate/Obj_FilterFlgCalculate/Long_ObjFilter/OverlapCalculate/BoudingBoxCalculate_Else/Overlap_FlgCalculate/AbsPosn_InsectionFlg/Overlap_Judgment/Overlap_FlgCalculate ==
  data: Obj_x_Max input; Overlap_Flg output; Obj_x_Min input; Obj_y_Max input; Obj_y_Min input; Subj_x_Max input; Subj_x_Min input; Subj_y_Max input; Subj_y_Min input
function Overlap_Flg = fcn(Obj_x_Max,Obj_x_Min,Obj_y_Max,Obj_y_Min,Subj_x_Max,Subj_x_Min,Subj_y_Max,Subj_y_Min)
if Obj_x_Max < Subj_x_Min || Obj_x_Min > Subj_x_Max
    Overlap_Flg = false;
else
    if Obj_y_Max < Subj_y_Min || Obj_y_Min > Subj_y_Max
        Overlap_Flg = false;
    else
        Overlap_Flg = true;
    end
end
end

== matlab function TosAeb/AEB_Objsel/TA_Processor/AEB_ObjSelect/TargetInfoCheck/Data_precess/TA_EffectCalculate/Obj_FilterFlgCalculate/Long_ObjFilter/OverlapCalculate/BoudingBoxCalculate_Else/PosnatTTI/PredictHostPositionAtTTR/CalculateHeading/ExtractVelocitiesAtStartOfSegments/ExtractVelocityVectorFirstPoint/PositionAndDerivative ==
  data: P0 input; x output single; P1 input; P2 input; P3 input; tau input; dx output single
function [x,dx] = fcn(P0,P1,P2,P3,tau)
%#codegen
[x, dx] = EstimatePositionAndDerivative(P0,P1,P2,P3,tau);

== matlab function TosAeb/AEB_Objsel/TA_Processor/AEB_ObjSelect/TargetInfoCheck/Data_precess/TA_EffectCalculate/Obj_FilterFlgCalculate/Long_ObjFilter/OverlapCalculate/BoudingBoxCalculate_Else/PosnatTTI/PredictHostPositionAtTTR/CalculateHeading/ExtractVelocitiesAtStartOfSegments/ExtractVelocityVectorFirstPoint/PositionAndDerivative1 ==
  data: P0 input; y output single; P1 input; P2 input; P3 input; tau input; dy output single
function [y,dy] = fcn(P0,P1,P2,P3,tau)
%#codegen

[y, dy] = EstimatePositionAndDerivative(P0,P1,P2,P3,tau);

== matlab function TosAeb/AEB_Objsel/TA_Processor/AEB_ObjSelect/TargetInfoCheck/Data_precess/TA_EffectCalculate/Obj_FilterFlgCalculate/Long_ObjFilter/OverlapCalculate/BoudingBoxCalculate_Else/PosnatTTI/PredictHostPositionAtTTR/CalculateHeading/FindValidIndex ==
  data: SegmentIndex input; SpeedValid input; ValidSegmentIndex output
function ValidSegmentIndex = fcn(SegmentIndex, SpeedValid)
%#codegen

% Searches for segment start points with detectable speed.
% The highest such index that is not further than the current segment is
% returned. Zero means that no such index were found.

% Default value of the found index.
ValidSegmentIndex = uint8(0);

for i = uint8(1:size(SpeedValid,1))
    % Loop through all indices and search for detectable speed.
    if SpeedValid(i) && i<=SegmentIndex
        % Speed is detectable and we have not searched further than the
        % current segment.
        ValidSegmentIndex = uint8(i);
    end
end
end

== matlab function TosAeb/AEB_Objsel/TA_Processor/AEB_ObjSelect/TargetInfoCheck/Data_precess/TA_EffectCalculate/Obj_FilterFlgCalculate/Long_ObjFilter/OverlapCalculate/BoudingBoxCalculate_Else/PosnatTTI/PredictHostPositionAtTTR/CalculateHeading/FindValidVelocityVector ==
  data: ValidVelocityY output; CurrentVelocityValid input; ValidVelocityX output; ValidSegmentIndex input; CurrentVelocityX input; CurrentVelocityY input; SegmentVelocitiesX input; SegmentVelocitiesY input
function [ValidVelocityY, ValidVelocityX] = fcn(CurrentVelocityValid, ValidSegmentIndex, CurrentVelocityX, CurrentVelocityY, SegmentVelocitiesX, SegmentVelocitiesY)
%#codegen

if CurrentVelocityValid
    % The current position in the segments has detectable speed.
    % Use that speed vector to estimate heading angle.
    ValidVelocityX = CurrentVelocityX;
    ValidVelocityY = CurrentVelocityY;
elseif ValidSegmentIndex > 0
    % An earlier start point of a segment has detectable speed.
    % Use the speed vector of the most recent start point with detectable
    % speed.
    ValidVelocityX = SegmentVelocitiesX(ValidSegmentIndex);
    ValidVelocityY = SegmentVelocitiesY(ValidSegmentIndex);
else
    % No valid speed has been detected.
    % Use speed vector that creates zero heading angle.
    % If we don't move, no heading change is needed.
    ValidVelocityX = single(0);
    ValidVelocityY = single(0);
end

end

== matlab function TosAeb/AEB_Objsel/TA_Processor/AEB_ObjSelect/TargetInfoCheck/Data_precess/TA_EffectCalculate/Obj_FilterFlgCalculate/Long_ObjFilter/OverlapCalculate/BoudingBoxCalculate_Else/PosnatTTI/PredictHostPositionAtTTR/LatPosition ==
  data: P0 input; y output single; P1 input; P2 input; P3 input; tau input; dy output single
function [y, dy] = fun(P0,P1,P2,P3,tau)
%#codegen

%TODO Description is missing 

%For performance reasons the original expression, below, has been rewritten  

%Original expressions 
% dx为x的对自变量tau的导数
% 式中x为距离，dx为速度
% 式中tau为x取值对应的概率值，p0，p1,p2,p3前系数值的和为"1"
%x=(1-tau)^3*P0 + 3*(1-tau)^2*tau*P1 + 3*(1-tau)*tau.^2*P2 + tau^3*P3;
%dx = -3*(1-tau)^2*P0 + 3*(3*tau^2 - 4*tau + 1)*P1 + 3*(2*tau - 3*tau^2)*P2 + 3*tau^2*P3; 

tauPov2 = tau*tau; 
tauPov3 = tau*tauPov2; 
x = (3*(P1 - P2) - P0 + P3)*tauPov3 + 3*(P0 - 2*P1 + P2)*tauPov2 + 3*(P1 - P0)*tau + P0; 
dx = (9*P1 - 3*P0 - 9*P2 + 3*P3)*tauPov2 + (6*P0 - 12*P1 + 6*P2)*tau - 3*P0 + 3*P1;
y = x;
dy = dx;

== matlab function TosAeb/AEB_Objsel/TA_Processor/AEB_ObjSelect/TargetInfoCheck/Data_precess/TA_EffectCalculate/Obj_FilterFlgCalculate/Long_ObjFilter/OverlapCalculate/BoudingBoxCalculate_Else/PosnatTTI/PredictHostPositionAtTTR/LatPosition1 ==
  data: P0 input; y output single; P1 input; P2 input; P3 input; tau input; dy output single
function [y, dy] = fun(P0,P1,P2,P3,tau)
%#codegen

%TODO Description is missing 

%For performance reasons the original expression, below, has been rewritten  

%Original expressions 
% dx为x的对自变量tau的导数
% 式中x为距离，dx为速度
% 式中tau为x取值对应的概率值，p0，p1,p2,p3前系数值的和为"1"
y=(1-tau)^3*P0 + 3*(1-tau)^2*tau*P1 + 3*(1-tau)*tau.^2*P2 + tau^3*P3;
dy = -3*(1-tau)^2*P0 + 3*(3*tau^2 - 4*tau + 1)*P1 + 3*(2*tau - 3*tau^2)*P2 + 3*tau^2*P3; 

%tauPov2 = tau*tau; 
%tauPov3 = tau*tauPov2; 
%x = (3*(P1 - P2) - P0 + P3)*tauPov3 + 3*(P0 - 2*P1 + P2)*tauPov2 + 3*(P1 - P0)*tau + P0; 
%dx = (9*P1 - 3*P0 - 9*P2 + 3*P3)*tauPov2 + (6*P0 - 12*P1 + 6*P2)*tau - 3*P0 + 3*P1;
%y = x;
%dy = dx;

== matlab function TosAeb/AEB_Objsel/TA_Processor/AEB_ObjSelect/TargetInfoCheck/Data_precess/TA_EffectCalculate/Obj_FilterFlgCalculate/Long_ObjFilter/OverlapCalculate/BoudingBoxCalculate_Else/PosnatTTI/PredictHostPositionAtTTR/LongPosition ==
  data: P0 input; x output single; P1 input; P2 input; P3 input; tau input; dx output single
function [x,dx] = fcn(P0,P1,P2,P3,tau)
%#codegen

%TODO Description is missing 

%For performance reasons the original expression, below, has been rewritten  

%Original expressions 
% dx为x的对自变量tau的导数
% 式中x为距离，dx为速度
% 式中tau为x取值对应的概率值，p0，p1,p2,p3前系数值的和为"1"
%x=(1-tau)^3*P0 + 3*(1-tau)^2*tau*P1 + 3*(1-tau)*tau.^2*P2 + tau^3*P3;
%dx = -3*(1-tau)^2*P0 + 3*(3*tau^2 - 4*tau + 1)*P1 + 3*(2*tau - 3*tau^2)*P2 + 3*tau^2*P3; 

tauPov2 = tau*tau; 
tauPov3 = tau*tauPov2; 
x = (3*(P1 - P2) - P0 + P3)*tauPov3 + 3*(P0 - 2*P1 + P2)*tauPov2 + 3*(P1 - P0)*tau + P0; 
dx = (9*P1 - 3*P0 - 9*P2 + 3*P3)*tauPov2 + (6*P0 - 12*P1 + 6*P2)*tau - 3*P0 + 3*P1;

== matlab function TosAeb/AEB_Objsel/TA_Processor/AEB_ObjSelect/TargetInfoCheck/Data_precess/TA_EffectCalculate/Obj_FilterFlgCalculate/Long_ObjFilter/OverlapCalculate/BoudingBoxCalculate_Else/PosnatTTI/PredictHostPositionAtTTR/LongPosition1 ==
  data: P0 input; x output single; P1 input; P2 input; P3 input; tau input; dx output single
function [x,dx] = fcn(P0,P1,P2,P3,tau)
%#codegen

%TODO Description is missing 

%For performance reasons the original expression, below, has been rewritten  

%Original expressions 
% dx为x的对自变量tau的导数
% 式中x为距离，dx为速度
% 式中tau为x取值对应的概率值，p0，p1,p2,p3前系数值的和为"1"
x=(1-tau)^3*P0 + 3*(1-tau)^2*tau*P1 + 3*(1-tau)*tau.^2*P2 + tau^3*P3;
dx = -3*(1-tau)^2*P0 + 3*(3*tau^2 - 4*tau + 1)*P1 + 3*(2*tau - 3*tau^2)*P2 + 3*tau^2*P3; 

%tauPov2 = tau*tau; 
%tauPov3 = tau*tauPov2; 
%x = (3*(P1 - P2) - P0 + P3)*tauPov3 + 3*(P0 - 2*P1 + P2)*tauPov2 + 3*(P1 - P0)*tau + P0; 
%dx = (9*P1 - 3*P0 - 9*P2 + 3*P3)*tauPov2 + (6*P0 - 12*P1 + 6*P2)*tau - 3*P0 + 3*P1;
